simulink model "curtain_brake"  (R2021a)
settings: Solver=ode23t  StartTime=0.0  StopTime=inf  RelTol=1e-3
model workspace (MATLAB code in the model file):
  | h = 1e-4; % Sampling time for decoder
  | PPR = 360; %
  | r_r=20e-3; %Radius of roller
  | a=5; % Height of curtain
  | b=4; % Width of curtain
  | c=2e-3; % Thickness of
  | g=9.8;
  | m_r=10; %mass of inner roller
  | m_w = 25/2*b; % mass of counter weights
  | f_c=1; %N/(m/s)/m friction of hanging curtain
  | f_r=0.1; %Nm/(rad/s) friction of roller
  | rho=1300 %kg/(m3) density of PVC
  | Td_B=8; %Derivative time constant for barrier damping
  | K_B = 100; % Gain for barrier
  | N_B = 1e3; % Max deriv gain for barrier
  | F_b = 50; %Nm max friction break torque
  | w_b = 1e-3; %Rad/sec when max friction torque reached
  | f_b = F_b/w_b; % Slope for linear part of friction model
  | V_b = 12; % Threshold voltage for releasing break
settings Model: EnablePacing=on  Creator=kjartan  LastModifiedBy=kjartan
settings SolverCC: AlgebraicLoopSolver=TrustRegion
settings DataIOCC: ReturnWorkspaceOutputs=off
settings DebuggingCC: SFMachineParentedDataDiag=warning  ArithmeticOperatorsInVariantConditions=warning
settings SFSimCC: CustomCodeUndefinedFunction=UseInterfaceOnly

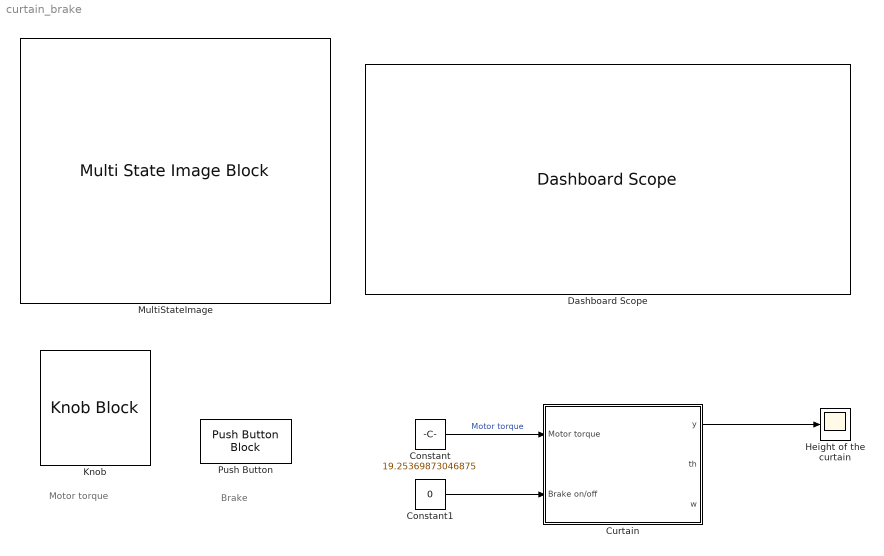
== system curtain_brake ==
  note: Motor torque
  note: Brake
  Constant : Constant  Value=19.25369873046875
  Constant1 : Constant  Value=0
  Curtain : Subsystem
  Dashboard Scope : DashboardScope  Ymin=-0.5  Ymax=6
  Height of the curtain : Scope
  Knob : KnobBlock  ScaleMax=40
  MultiStateImage : MultiStateImageBlock
  Push Button : PushButtonBlock
  wires:
  Constant/1 -> Curtain/1(Motor torque)  "Motor torque"
  Curtain/1(y) -> Height of the curtain/1
  Constant1/1 -> Curtain/2(Brake on/off)

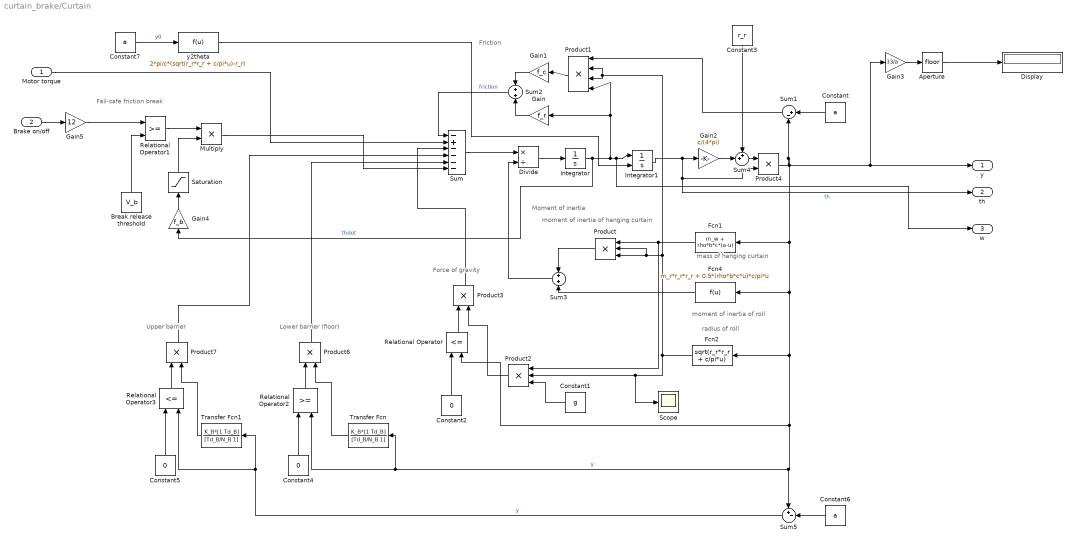
== system curtain_brake/Curtain ==
  note: Fail-safe friction break
  note: Force of gravity
  note: Moment of inertia
  note: Friction
  note: Lower barrier (floor)
  note: moment of inertia of hanging curtain
  note: moment of inertia of roll
  note: radius of roll
  note: mass of hanging curtain
  note: Upper barrier
  Motor torque : Inport
  Brake on/off : Inport  Port=2
  Aperture : Rounding
  Break release threshold : Constant  Value=V_b
  Constant : Constant  Value=a
  Constant1 : Constant  Value=g
  Constant2 : Constant  Value=0
  Constant3 : Constant  Value=r_r
  Constant4 : Constant  Value=0
  Constant5 : Constant  Value=0
  Constant6 : Constant  Value=a
  Constant7 : Constant  Value=a
  Display : Display
  Divide : Product  Inputs=*/
  Fcn1 : Fcn  Expr=m_w + rho*b*c*(a-u)
  Fcn2 : Fcn  Expr=sqrt(r_r*r_r + c/pi*u)
  Fcn4 : Fcn  Expr=m_r*r_r*r_r + 0.5*(rho*b*c*u)*c/pi*u
  Gain : Gain  Gain=f_r
  Gain1 : Gain  Gain=f_c
  Gain2 : Gain  Gain=c/(4*pi)
  Gain3 : Gain  Gain=33/a
  Gain4 : Gain  Gain=f_b
  Gain5 : Gain  Gain=12
  Integrator : Integrator
  Integrator1 : Integrator  InitialConditionSource=external  InitialCondition=12*2*pi
  Multiply : Product
  Product : Product  Inputs=3
  Product1 : Product  Inputs=4
  Product2 : Product  Inputs=3
  Product{3..4,6..7} (4 alike) : Product
  Relational Operator : RelationalOperator  Operator=<=  OutDataTypeStr=boolean  RndMeth=Simplest
  Relational Operator1 : RelationalOperator  OutDataTypeStr=boolean  RndMeth=Simplest
  Relational Operator2 : RelationalOperator  OutDataTypeStr=boolean  RndMeth=Simplest
  Relational Operator3 : RelationalOperator  Operator=<=  OutDataTypeStr=boolean  RndMeth=Simplest
  Saturation : Saturate  UpperLimit=F_b  LowerLimit=-F_b
  Scope : Scope
  Sum : Sum  Inputs=-+----
  Sum1 : Sum  Inputs=|+-
  Sum2 : Sum  Inputs=+|+
  Sum3 : Sum  Inputs=+|+
  Sum4 : Sum  Inputs=++|
  Sum5 : Sum  Inputs=+-|
  Transfer Fcn : TransferFcn  Numerator=K_B*[1 Td_B]  Denominator=[Td_B/N_B 1]
  Transfer Fcn1 : TransferFcn  Numerator=K_B*[1 Td_B]  Denominator=[Td_B/N_B 1]
  y2theta : Fcn  Expr=2*pi/c*(sqrt(r_r*r_r + c/pi*u)-r_r)
  y : Outport  VectorParamsAs1DForOutWhenUnconnected=off
  th : Outport  Port=2  VectorParamsAs1DForOutWhenUnconnected=off
  w : Outport  Port=3
  wires:
  Relational Operator/1 -> Product3/1
  Fcn4/1 -> Sum3/2
  Constant/1 -> Sum1/1
  Gain/1 -> Sum2/2
  Constant1/1 -> Product2/3
  Product2/1 -> Product3/2
  Relational Operator3/1 -> Product7/1
  Divide/1 -> Integrator/1
  Sum4/1 -> Product4/1
  Constant3/1 -> Sum4/1
  Constant4/1 -> Relational Operator2/1
  Constant6/1 -> Sum5/2
  Sum5/1 -> Transfer Fcn1/1, Relational Operator3/2  "y"
  Fcn1/1 -> Product2/1, Product/1
  Product4/1 -> Gain3/1, y/1, Sum5/1, Transfer Fcn/1, Relational Operator2/2, Relational Operator/2, Fcn2/1, Fcn4/1, Fcn1/1, Sum1/2  "y"
  Constant2/1 -> Relational Operator/1
  Integrator1/1 -> th/1, Product4/2, Gain2/1  "th"
  Sum2/1 -> Sum/1  "friction"
  Fcn2/1 -> Product1/2, Product1/3, Product/2, Product/3, Scope/1, Product2/2
  Motor torque/1 -> Sum/2
  Gain1/1 -> Sum2/1
  Product/1 -> Sum3/1
  Sum3/1 -> Divide/2
  Relational Operator2/1 -> Product6/1
  Integrator/1 -> Gain4/1, Product1/4, Gain/1, w/1, Integrator1/1  "thdot"
  Product7/1 -> Sum/4
  Product1/1 -> Gain1/1
  Constant5/1 -> Relational Operator3/1
  Sum1/1 -> Product1/1
  Product6/1 -> Sum/5
  Product3/1 -> Sum/3
  Transfer Fcn/1 -> Product6/2
  Sum/1 -> Divide/1
  Gain2/1 -> Sum4/2
  Transfer Fcn1/1 -> Product7/2
  Gain3/1 -> Aperture/1
  Aperture/1 -> Display/1
  Constant7/1 -> y2theta/1  "y0"
  y2theta/1 -> Integrator1/2
  Brake on/off/1 -> Gain5/1
  Break release threshold/1 -> Relational Operator1/2
  Relational Operator1/1 -> Multiply/1
  Gain4/1 -> Saturation/1
  Saturation/1 -> Multiply/2
  Multiply/1 -> Sum/6
  Gain5/1 -> Relational Operator1/1
